simulink model "CCMHBMFMgr"  (R2022b)
settings: Solver=FixedStepDiscrete  StartTime=0.0  StopTime=10.0  FixedStep=0.1  RelTol=1e-3
undefined symbols (not defined in the file or in its folder): NetworkChlType, P_LinIfSchHandleType
settings Model: Creator=blue.ge  LastModifiedBy=blue.ge
settings DataIOCC: SaveFormat=StructureWithTime
settings OptimizationCC: DefaultParameterBehavior=Inlined  EnhancedBackFolding=on  PassReuseOutputArgsAs=Individual arguments  ZeroExternalMemoryAtStartup=off  ZeroInternalMemoryAtStartup=off  OptimizeBlockOrder=speed  DifferentSizesBufferReuse=on  OptimizationCustomize=off  EfficientTunableParamExpr=on
settings DebuggingCC: ParameterTunabilityLossMsg=error
settings ModelReferenceCC: ModelReferenceNumInstancesAllowed=Single
settings SFSimCC: MATLABDynamicMemAlloc=off
settings RTWCC: Description=AUTOSAR  SystemTargetFile=autosar.tlc  GenCodeOnly=on  TemplateMakefile=ert_default_tmf
settings CodeAppCC: ForceParamTrailComments=on  IgnoreCustomStorageClasses=off  OperatorAnnotations=on  SimulinkDataObjDesc=on  SFDataObjDesc=on  InsertBlockDesc=on
settings STFCustomTargetCC: TargetLangStandard=C99 (ISO)  MultiwordLength=256  ParMdlRefBuildCompliant=on  CompOptLevelCompliant=on  IncludeMdlTerminateFcn=off  CombineOutputUpdateFcns=on  SuppressErrorStatus=on  ERTCustomFileBanners=on  SupportNonFinite=off  SupportComplex=off  SupportContinuousTime=off  SupportNonInlinedSFcns=off  RemoveResetFunc=on  MATLABClassNameForMDSCustomization=Simulink.SoftwareTarget.ERTCustomization  AutosarCompliant=on  UseToolchainInfoCompliant=on  LookupTableObjectStructAxisOrder=2,1,3,4,...  LUTObjectStructOrderEvenSpacing=Size,Table,Breakpoints  SystemTargetFile=autosar.tlc
settings ERTTargetCC: TargetLangStandard=C99 (ISO)  ConcurrentExecutionCompliant=off  IncludeMdlTerminateFcn=off  SuppressErrorStatus=on  SupportNonFinite=off  SupportComplex=off  ModelStepFunctionPrototypeControlCompliant=off  CPPClassGenCompliant=off  AutosarCompliant=on  LookupTableObjectStructAxisOrder=2,1,3,4,...  LUTObjectStructOrderEvenSpacing=Size,Table,Breakpoints  InlinedParameterPlacement=NonHierarchical  ERTCustomFileTemplate=example_file_process.tlc

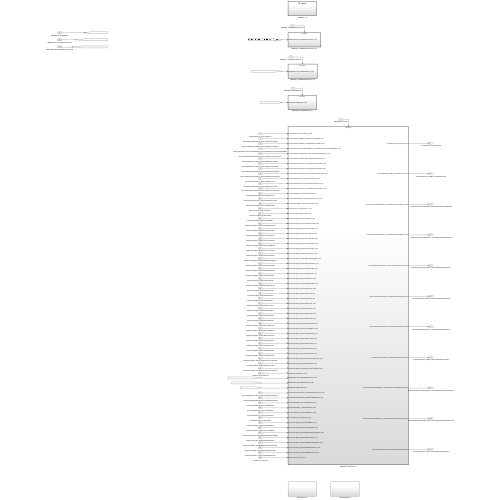
[thumbnail: Simulink's stored preview of the top-level diagram]
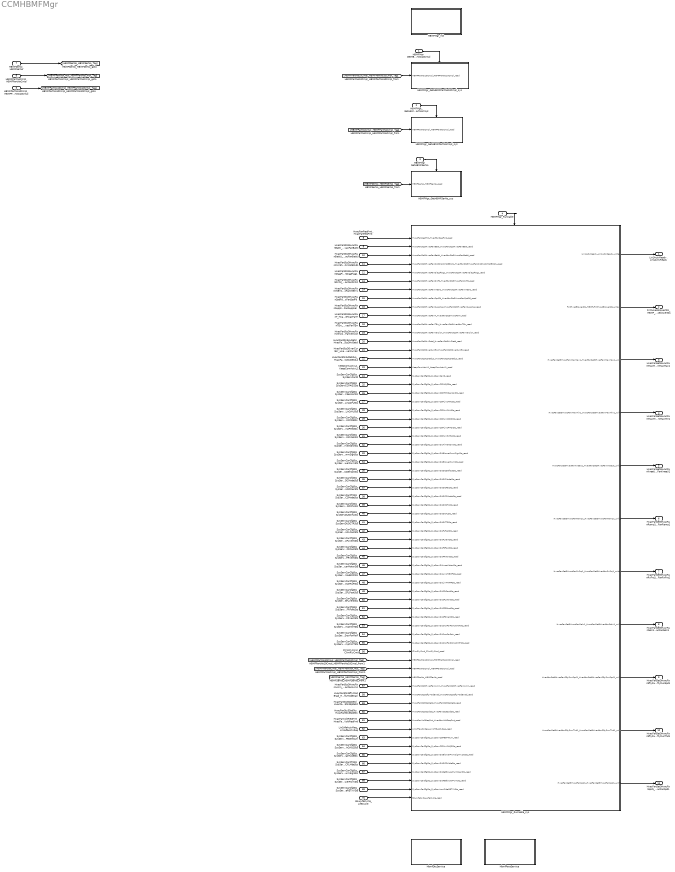
== system CCMHBMFMgr ==
  HBMFMgr_GetHBMFPartNo10Cmpl : Inport  OutputFunctionCall=on
  HBMFMgr_GetHBMFPartNoCmpl : Inport  Port=2  OutputFunctionCall=on
  HBMFMgr_GetHBMFSerNo : Inport  Port=3  OutputFunctionCall=on
  HBMFMgr_Runnable : Inport  Port=4  SampleTime=0.1  OutputFunctionCall=on
  HBMFPartNo10Cmpl_HBMFPartNo10Cmpl : Inport  Port=5  OutDataTypeStr=Bus: PartNrNod10  PortDimensions=1  BusOutputAsStruct=on
  HBMFPartNoCmpl_HBMFPartNoCmpl : Inport  Port=6  OutDataTypeStr=Bus: PartNrNodLIN  PortDimensions=1  BusOutputAsStruct=on
  HBMFSerNo_HBMFSerNo : Inport  Port=7  OutDataTypeStr=Bus: NrSerlNodLIN  PortDimensions=1  BusOutputAsStruct=on
  HvacFanReqFrnt_HvacFanReqFrnt : Inport  Port=8  OutDataTypeStr=HvacFanSpd  PortDimensions=1
  HvacFanSts3HvacFanBattI_HvacFanSts3HvacFanBattI : Inport  Port=9  OutDataTypeStr=BlowerBattI  PortDimensions=1
  HvacFanSts3HvacFanBattU_HvacFanSts3HvacFanBattU : Inport  Port=10  OutDataTypeStr=U10  PortDimensions=1
  HvacFanSts3HvacFanCircShoOrMotBlkd1_HvacFanSts3HvacFanCircShoOrMotBlkd1 : Inport  Port=11  OutDataTypeStr=HvacFanCircShoOrMotBlkdTyp  PortDimensions=1
  HvacFanSts3HvacFanDiagFlag1_HvacFanSts3HvacFanDiagFlag1 : Inport  Port=12  OutDataTypeStr=Enum: HvacFanDiagFlag  PortDimensions=1
  HvacFanSts3HvacFanDirFd_HvacFanSts3HvacFanDirFd : Inport  Port=13  OutDataTypeStr=Enum: HvacFanDir  PortDimensions=1
  HvacFanSts3HvacFanIntErr1_HvacFanSts3HvacFanIntErr1 : Inport  Port=14  OutDataTypeStr=HvacFanIntErrTyp  PortDimensions=1
  HvacFanSts3HvacFanSpdFd_HvacFanSts3HvacFanSpdFd : Inport  Port=15  OutDataTypeStr=BlowerSpd  PortDimensions=1
  HvacFanSts3HvacFanSupplier_HvacFanSts3HvacFanSupplier : Inport  Port=16  OutMin=0  OutMax=15  OutDataTypeStr=UInt4  PortDimensions=1
  HvacFanSts3HvacFanT_HvacFanSts3HvacFanT : Inport  Port=17  OutDataTypeStr=T6  PortDimensions=1
  HvacFanSts3HvacFanTErr_HvacFanSts3HvacFanTErr : Inport  Port=18  OutDataTypeStr=Boolean  PortDimensions=1
  HvacFanSts3HvacFanVersion_HvacFanSts3HvacFanVersion : Inport  Port=19  OutDataTypeStr=UInt8  PortDimensions=1
  HvacFanSts3HvDetd_HvacFanSts3HvDetd : Inport  Port=20  OutDataTypeStr=Boolean  PortDimensions=1
  HvacFanSts3OverCurrErr_HvacFanSts3OverCurrErr : Inport  Port=21  OutDataTypeStr=Boolean  PortDimensions=1
  HvacFanSts3UDetdLo_HvacFanSts3UDetdLo : Inport  Port=22  OutDataTypeStr=Boolean  PortDimensions=1
  KeepComActvL1_KeepComActvL1 : Inport  Port=23  OutDataTypeStr=Enum: OnOff1  PortDimensions=1
  SysServCarCfgSts_SysServCarID : Inport  Port=24  OutDataTypeStr=Enum: SysServCarID  PortDimensions=1
  SysServCarCfgSts_SysServCCPAQSSts : Inport  Port=25  OutDataTypeStr=UInt8  PortDimensions=1
  SysServCarCfgSts_SysServCCPHEXSensorSts : Inport  Port=26  OutDataTypeStr=UInt8  PortDimensions=1
  SysServCarCfgSts_SysServCCPLin2AFUSts : Inport  Port=27  OutDataTypeStr=Boolean  PortDimensions=1
  SysServCarCfgSts_SysServCCPLin2AIUSts : Inport  Port=28  OutDataTypeStr=UInt8  PortDimensions=1
  SysServCarCfgSts_SysServCCPLin2CDSSts : Inport  Port=29  OutDataTypeStr=Boolean  PortDimensions=1
  SysServCarCfgSts_SysServCCPLin2PMSISts : Inport  Port=30  OutDataTypeStr=Boolean  PortDimensions=1
  SysServCarCfgSts_SysServCCPLin3VGASts : Inport  Port=31  OutDataTypeStr=Boolean  PortDimensions=1
  SysServCarCfgSts_SysServDUAirVentSnrSts : Inport  Port=32  OutDataTypeStr=Boolean  PortDimensions=1
  SysServCarCfgSts_SysServDUBlowerPwmSignSts : Inport  Port=33  OutDataTypeStr=Boolean  PortDimensions=1
  SysServCarCfgSts_SysServDUBlowerPwmSts : Inport  Port=34  OutDataTypeStr=Boolean  PortDimensions=1
  SysServCarCfgSts_SysServDUDCDefrostSts : Inport  Port=35  OutDataTypeStr=UInt8  PortDimensions=1
  SysServCarCfgSts_SysServDUDCModeSts : Inport  Port=36  OutDataTypeStr=UInt8  PortDimensions=1
  SysServCarCfgSts_SysServDUDCRecSts : Inport  Port=37  OutDataTypeStr=UInt8  PortDimensions=1
  SysServCarCfgSts_SysServDUDCSModeSts : Inport  Port=38  OutDataTypeStr=UInt8  PortDimensions=1
  SysServCarCfgSts_SysServDUDCSTLSts : Inport  Port=39  OutDataTypeStr=UInt8  PortDimensions=1
  SysServCarCfgSts_SysServDUDCTLSts : Inport  Port=40  OutDataTypeStr=UInt8  PortDimensions=1
  SysServCarCfgSts_SysServDUDCTRSts : Inport  Port=41  OutDataTypeStr=UInt8  PortDimensions=1
  SysServCarCfgSts_SysServDUFLFootSts : Inport  Port=42  OutDataTypeStr=UInt8  PortDimensions=1
  SysServCarCfgSts_SysServDUFLVentSts : Inport  Port=43  OutDataTypeStr=UInt8  PortDimensions=1
  SysServCarCfgSts_SysServDUFRFootSts : Inport  Port=44  OutDataTypeStr=UInt8  PortDimensions=1
  SysServCarCfgSts_SysServDUFRVentSts : Inport  Port=45  OutDataTypeStr=UInt8  PortDimensions=1
  SysServCarCfgSts_SysServDUIncarMotorSts : Inport  Port=46  OutDataTypeStr=Boolean  PortDimensions=1
  SysServCarCfgSts_SysServDULin1HBMFSts : Inport  Port=47  OutDataTypeStr=Boolean  PortDimensions=1
  SysServCarCfgSts_SysServDULin4HFAFSts : Inport  Port=48  OutDataTypeStr=UInt32  PortDimensions=1
  SysServCarCfgSts_SysServDUSFLFootSts : Inport  Port=49  OutDataTypeStr=UInt8  PortDimensions=1
  SysServCarCfgSts_SysServDUSFLVentSts : Inport  Port=50  OutDataTypeStr=UInt8  PortDimensions=1
  SysServCarCfgSts_SysServDUSFRFootSts : Inport  Port=51  OutDataTypeStr=UInt8  PortDimensions=1
  SysServCarCfgSts_SysServDUSFRVentSts : Inport  Port=52  OutDataTypeStr=UInt8  PortDimensions=1
  SysServCarCfgSts_SysServDUSnsrForFCmptmtTSts : Inport  Port=53  OutDataTypeStr=UInt8  PortDimensions=1
  SysServCarCfgSts_SysServDUSnsrForRair : Inport  Port=54  OutDataTypeStr=UInt8  PortDimensions=1
  SysServCarCfgSts_SysServDUSnsrForRCmptmtTSts : Inport  Port=55  OutDataTypeStr=UInt8  PortDimensions=1
  ClimRlyCmd_ClimRlyCmd : Inport  Port=56  OutDataTypeStr=Enum: OnOff1  PortDimensions=1
  HvacFanSts3HvacFanILimn_HvacFanSts3HvacFanILimn : Inport  Port=57  OutDataTypeStr=Boolean  PortDimensions=1
  HvacFanSts3SftyModEnad_HvacFanSts3SftyModEnad : Inport  Port=58  OutDataTypeStr=Boolean  PortDimensions=1
  HvacFanSts3SpDetd_HvacFanSts3SpDetd : Inport  Port=59  OutDataTypeStr=Boolean  PortDimensions=1
  HvacFanSts3SpdSts_HvacFanSts3SpdSts : Inport  Port=60  OutDataTypeStr=Boolean  PortDimensions=1
  HvacFanVoltReqFrnt_HvacFanVoltReqFrnt : Inport  Port=61  OutMin=0  OutMax=100  OutDataTypeStr=Perc9  PortDimensions=1
  Lin1HfaActvReq_Lin1HfaActvReq : Inport  Port=62  OutDataTypeStr=UInt8  PortDimensions=1
  SysServCarCfgSts_SysServCCPBGMNum : Inport  Port=63  OutDataTypeStr=UInt8  PortDimensions=1
  SysServCarCfgSts_SysServCCPLin2AQSSts : Inport  Port=64  OutDataTypeStr=Boolean  PortDimensions=1
  SysServCarCfgSts_SysServDUBlowerPwmSignTypeSts : Inport  Port=65  OutDataTypeStr=UInt8  PortDimensions=1
  SysServCarCfgSts_SysServDUDCFLMdeSts : Inport  Port=66  OutDataTypeStr=UInt8  PortDimensions=1
  SysServCarCfgSts_SysServDUReBlowerPwmSignSts : Inport  Port=67  OutDataTypeStr=UInt8  PortDimensions=1
  SysServCarCfgSts_SysServDUReBlowerPwmSts : Inport  Port=68  OutDataTypeStr=UInt8  PortDimensions=1
  SysServCarCfgSts_SysServLowSideRETXVSts : Inport  Port=69  OutDataTypeStr=UInt8  PortDimensions=1
  BswLifeCycle_LifeCycle : Inport  Port=70  OutDataTypeStr=Enum: CCMLifeCycleModeGroup  PortDimensions=1
  HBMFMgr_GetHBMFPartNo10Cmpl_sys : Subsystem
  HBMFMgr_GetHBMFPartNoCmpl_sys : Subsystem
  HBMFMgr_GetHBMFSerNo_sys : Subsystem
  HBMFMgr_Init : Subsystem
  HBMFMgr_Runnable_sys : Subsystem
  HbmfDtcService : Subsystem
  HbmfParaService : Subsystem
  Lin1ActvReady_Lin1ActvReady : Outport  OutDataTypeStr=UInt8  PortDimensions=1
  FrntHvacBlowerSts_HBMF_FrntHvacBlowerSts : Outport  Port=2  OutDataTypeStr=Enum: HvacFanSts  PortDimensions=1
  HvacFanSet3HvacFanIMaxMax1_HvacFanSet3HvacFanIMaxMax1 : Outport  Port=3  OutDataTypeStr=BlowerIMAX1  PortDimensions=1
  HvacFanSet3HvacFanIMaxMin1_HvacFanSet3HvacFanIMaxMin1 : Outport  Port=4  OutDataTypeStr=Ampr3  PortDimensions=1
  HvacFanSet3HvacFanKneeU1_HvacFanSet3HvacFanKneeU1 : Outport  Port=5  OutDataTypeStr=U53  PortDimensions=1
  HvacFanSet3HvacFanRamp1_HvacFanSet3HvacFanRamp1 : Outport  Port=6  OutDataTypeStr=BlowerRamp  PortDimensions=1
  HvacFanSet3HvacFanRxFrq1_HvacFanSet3HvacFanRxFrq1 : Outport  Port=7  OutDataTypeStr=Uint12  PortDimensions=1
  HvacFanSet3HvacFanSeln1_HvacFanSet3HvacFanSeln1 : Outport  Port=8  OutDataTypeStr=Enum: HvacFanSeln  PortDimensions=1
  HvacFanSet3HvacFanSftyRunSpd1_HvacFanSet3HvacFanSftyRunSpd1 : Outport  Port=9  OutDataTypeStr=BlowerSpd  PortDimensions=1
  HvacFanSet3HvacFanSftyRunThd1_HvacFanSet3HvacFanSftyRunThd1 : Outport  Port=10  OutDataTypeStr=HvacFanSftyRunThd  PortDimensions=1
  HvacFanSet3HvacFanSpd1_HvacFanSet3HvacFanSpd1 : Outport  Port=11  OutDataTypeStr=BlowerSpd  PortDimensions=1
  wires:
  HvacFanReqFrnt_HvacFanReqFrnt/1 -> HBMFMgr_Runnable_sys/1(HvacFanReqFrnt_HvacFanReqFrnt_read)
  HvacFanSts3HvacFanBattI_HvacFanSts3HvacFanBattI/1 -> HBMFMgr_Runnable_sys/2(HvacFanSts3HvacFanBattI_HvacFanSts3HvacFanBattI_read)
  HvacFanSts3HvacFanBattU_HvacFanSts3HvacFanBattU/1 -> HBMFMgr_Runnable_sys/3(HvacFanSts3HvacFanBattU_HvacFanSts3HvacFanBattU_read)
  HvacFanSts3HvacFanCircShoOrMotBlkd1_HvacFanSts3HvacFanCircShoOrMotBlkd1/1 -> HBMFMgr_Runnable_sys/4(HvacFanSts3HvacFanCircShoOrMotBlkd1_HvacFanSts3HvacFanCircShoOrMotBlkd1_read)
  HvacFanSts3HvacFanDiagFlag1_HvacFanSts3HvacFanDiagFlag1/1 -> HBMFMgr_Runnable_sys/5(HvacFanSts3HvacFanDiagFlag1_HvacFanSts3HvacFanDiagFlag1_read)
  HvacFanSts3HvacFanDirFd_HvacFanSts3HvacFanDirFd/1 -> HBMFMgr_Runnable_sys/6(HvacFanSts3HvacFanDirFd_HvacFanSts3HvacFanDirFd_read)
  HvacFanSts3HvacFanIntErr1_HvacFanSts3HvacFanIntErr1/1 -> HBMFMgr_Runnable_sys/7(HvacFanSts3HvacFanIntErr1_HvacFanSts3HvacFanIntErr1_read)
  HvacFanSts3HvacFanSpdFd_HvacFanSts3HvacFanSpdFd/1 -> HBMFMgr_Runnable_sys/8(HvacFanSts3HvacFanSpdFd_HvacFanSts3HvacFanSpdFd_read)
  HvacFanSts3HvacFanSupplier_HvacFanSts3HvacFanSupplier/1 -> HBMFMgr_Runnable_sys/9(HvacFanSts3HvacFanSupplier_HvacFanSts3HvacFanSupplier_read)
  HvacFanSts3HvacFanT_HvacFanSts3HvacFanT/1 -> HBMFMgr_Runnable_sys/10(HvacFanSts3HvacFanT_HvacFanSts3HvacFanT_read)
  HvacFanSts3HvacFanTErr_HvacFanSts3HvacFanTErr/1 -> HBMFMgr_Runnable_sys/11(HvacFanSts3HvacFanTErr_HvacFanSts3HvacFanTErr_read)
  HvacFanSts3HvacFanVersion_HvacFanSts3HvacFanVersion/1 -> HBMFMgr_Runnable_sys/12(HvacFanSts3HvacFanVersion_HvacFanSts3HvacFanVersion_read)
  HvacFanSts3HvDetd_HvacFanSts3HvDetd/1 -> HBMFMgr_Runnable_sys/13(HvacFanSts3HvDetd_HvacFanSts3HvDetd_read)
  HvacFanSts3OverCurrErr_HvacFanSts3OverCurrErr/1 -> HBMFMgr_Runnable_sys/14(HvacFanSts3OverCurrErr_HvacFanSts3OverCurrErr_read)
  HvacFanSts3UDetdLo_HvacFanSts3UDetdLo/1 -> HBMFMgr_Runnable_sys/15(HvacFanSts3UDetdLo_HvacFanSts3UDetdLo_read)
  KeepComActvL1_KeepComActvL1/1 -> HBMFMgr_Runnable_sys/16(KeepComActvL1_KeepComActvL1_read)
  SysServCarCfgSts_SysServCarID/1 -> HBMFMgr_Runnable_sys/17(SysServCarCfgSts_SysServCarID_read)
  SysServCarCfgSts_SysServCCPAQSSts/1 -> HBMFMgr_Runnable_sys/18(SysServCarCfgSts_SysServCCPAQSSts_read)
  SysServCarCfgSts_SysServCCPHEXSensorSts/1 -> HBMFMgr_Runnable_sys/19(SysServCarCfgSts_SysServCCPHEXSensorSts_read)
  SysServCarCfgSts_SysServCCPLin2AFUSts/1 -> HBMFMgr_Runnable_sys/20(SysServCarCfgSts_SysServCCPLin2AFUSts_read)
  SysServCarCfgSts_SysServCCPLin2AIUSts/1 -> HBMFMgr_Runnable_sys/21(SysServCarCfgSts_SysServCCPLin2AIUSts_read)
  SysServCarCfgSts_SysServCCPLin2CDSSts/1 -> HBMFMgr_Runnable_sys/22(SysServCarCfgSts_SysServCCPLin2CDSSts_read)
  SysServCarCfgSts_SysServCCPLin2PMSISts/1 -> HBMFMgr_Runnable_sys/23(SysServCarCfgSts_SysServCCPLin2PMSISts_read)
  SysServCarCfgSts_SysServCCPLin3VGASts/1 -> HBMFMgr_Runnable_sys/24(SysServCarCfgSts_SysServCCPLin3VGASts_read)
  SysServCarCfgSts_SysServDUAirVentSnrSts/1 -> HBMFMgr_Runnable_sys/25(SysServCarCfgSts_SysServDUAirVentSnrSts_read)
  SysServCarCfgSts_SysServDUBlowerPwmSignSts/1 -> HBMFMgr_Runnable_sys/26(SysServCarCfgSts_SysServDUBlowerPwmSignSts_read)
  SysServCarCfgSts_SysServDUBlowerPwmSts/1 -> HBMFMgr_Runnable_sys/27(SysServCarCfgSts_SysServDUBlowerPwmSts_read)
  SysServCarCfgSts_SysServDUDCDefrostSts/1 -> HBMFMgr_Runnable_sys/28(SysServCarCfgSts_SysServDUDCDefrostSts_read)
  SysServCarCfgSts_SysServDUDCModeSts/1 -> HBMFMgr_Runnable_sys/29(SysServCarCfgSts_SysServDUDCModeSts_read)
  SysServCarCfgSts_SysServDUDCRecSts/1 -> HBMFMgr_Runnable_sys/30(SysServCarCfgSts_SysServDUDCRecSts_read)
  SysServCarCfgSts_SysServDUDCSModeSts/1 -> HBMFMgr_Runnable_sys/31(SysServCarCfgSts_SysServDUDCSModeSts_read)
  SysServCarCfgSts_SysServDUDCSTLSts/1 -> HBMFMgr_Runnable_sys/32(SysServCarCfgSts_SysServDUDCSTLSts_read)
  SysServCarCfgSts_SysServDUDCTLSts/1 -> HBMFMgr_Runnable_sys/33(SysServCarCfgSts_SysServDUDCTLSts_read)
  SysServCarCfgSts_SysServDUDCTRSts/1 -> HBMFMgr_Runnable_sys/34(SysServCarCfgSts_SysServDUDCTRSts_read)
  SysServCarCfgSts_SysServDUFLFootSts/1 -> HBMFMgr_Runnable_sys/35(SysServCarCfgSts_SysServDUFLFootSts_read)
  SysServCarCfgSts_SysServDUFLVentSts/1 -> HBMFMgr_Runnable_sys/36(SysServCarCfgSts_SysServDUFLVentSts_read)
  SysServCarCfgSts_SysServDUFRFootSts/1 -> HBMFMgr_Runnable_sys/37(SysServCarCfgSts_SysServDUFRFootSts_read)
  SysServCarCfgSts_SysServDUFRVentSts/1 -> HBMFMgr_Runnable_sys/38(SysServCarCfgSts_SysServDUFRVentSts_read)
  SysServCarCfgSts_SysServDUIncarMotorSts/1 -> HBMFMgr_Runnable_sys/39(SysServCarCfgSts_SysServDUIncarMotorSts_read)
  SysServCarCfgSts_SysServDULin1HBMFSts/1 -> HBMFMgr_Runnable_sys/40(SysServCarCfgSts_SysServDULin1HBMFSts_read)
  SysServCarCfgSts_SysServDULin4HFAFSts/1 -> HBMFMgr_Runnable_sys/41(SysServCarCfgSts_SysServDULin4HFAFSts_read)
  SysServCarCfgSts_SysServDUSFLFootSts/1 -> HBMFMgr_Runnable_sys/42(SysServCarCfgSts_SysServDUSFLFootSts_read)
  SysServCarCfgSts_SysServDUSFLVentSts/1 -> HBMFMgr_Runnable_sys/43(SysServCarCfgSts_SysServDUSFLVentSts_read)
  SysServCarCfgSts_SysServDUSFRFootSts/1 -> HBMFMgr_Runnable_sys/44(SysServCarCfgSts_SysServDUSFRFootSts_read)
  SysServCarCfgSts_SysServDUSFRVentSts/1 -> HBMFMgr_Runnable_sys/45(SysServCarCfgSts_SysServDUSFRVentSts_read)
  SysServCarCfgSts_SysServDUSnsrForFCmptmtTSts/1 -> HBMFMgr_Runnable_sys/46(SysServCarCfgSts_SysServDUSnsrForFCmptmtTSts_read)
  SysServCarCfgSts_SysServDUSnsrForRair/1 -> HBMFMgr_Runnable_sys/47(SysServCarCfgSts_SysServDUSnsrForRair_read)
  SysServCarCfgSts_SysServDUSnsrForRCmptmtTSts/1 -> HBMFMgr_Runnable_sys/48(SysServCarCfgSts_SysServDUSnsrForRCmptmtTSts_read)
  ClimRlyCmd_ClimRlyCmd/1 -> HBMFMgr_Runnable_sys/49(ClimRlyCmd_ClimRlyCmd_read)
  HvacFanSts3HvacFanILimn_HvacFanSts3HvacFanILimn/1 -> HBMFMgr_Runnable_sys/53(HvacFanSts3HvacFanILimn_HvacFanSts3HvacFanILimn_read)
  HvacFanSts3SftyModEnad_HvacFanSts3SftyModEnad/1 -> HBMFMgr_Runnable_sys/54(HvacFanSts3SftyModEnad_HvacFanSts3SftyModEnad_read)
  HvacFanSts3SpDetd_HvacFanSts3SpDetd/1 -> HBMFMgr_Runnable_sys/55(HvacFanSts3SpDetd_HvacFanSts3SpDetd_read)
  HvacFanSts3SpdSts_HvacFanSts3SpdSts/1 -> HBMFMgr_Runnable_sys/56(HvacFanSts3SpdSts_HvacFanSts3SpdSts_read)
  HvacFanVoltReqFrnt_HvacFanVoltReqFrnt/1 -> HBMFMgr_Runnable_sys/57(HvacFanVoltReqFrnt_HvacFanVoltReqFrnt_read)
  Lin1HfaActvReq_Lin1HfaActvReq/1 -> HBMFMgr_Runnable_sys/58(Lin1HfaActvReq_Lin1HfaActvReq_read)
  SysServCarCfgSts_SysServCCPBGMNum/1 -> HBMFMgr_Runnable_sys/59(SysServCarCfgSts_SysServCCPBGMNum_read)
  SysServCarCfgSts_SysServCCPLin2AQSSts/1 -> HBMFMgr_Runnable_sys/60(SysServCarCfgSts_SysServCCPLin2AQSSts_read)
  SysServCarCfgSts_SysServDUBlowerPwmSignTypeSts/1 -> HBMFMgr_Runnable_sys/61(SysServCarCfgSts_SysServDUBlowerPwmSignTypeSts_read)
  SysServCarCfgSts_SysServDUDCFLMdeSts/1 -> HBMFMgr_Runnable_sys/62(SysServCarCfgSts_SysServDUDCFLMdeSts_read)
  SysServCarCfgSts_SysServDUReBlowerPwmSignSts/1 -> HBMFMgr_Runnable_sys/63(SysServCarCfgSts_SysServDUReBlowerPwmSignSts_read)
  SysServCarCfgSts_SysServDUReBlowerPwmSts/1 -> HBMFMgr_Runnable_sys/64(SysServCarCfgSts_SysServDUReBlowerPwmSts_read)
  SysServCarCfgSts_SysServLowSideRETXVSts/1 -> HBMFMgr_Runnable_sys/65(SysServCarCfgSts_SysServLowSideRETXVSts_read)
  HBMFMgr_Runnable_sys/1(Lin1ActvReady_Lin1ActvReady_write) -> Lin1ActvReady_Lin1ActvReady/1
  HBMFMgr_Runnable_sys/2(FrntHvacBlowerSts_HBMF_FrntHvacBlowerSts_write) -> FrntHvacBlowerSts_HBMF_FrntHvacBlowerSts/1
  HBMFMgr_Runnable_sys/3(HvacFanSet3HvacFanIMaxMax1_HvacFanSet3HvacFanIMaxMax1_write) -> HvacFanSet3HvacFanIMaxMax1_HvacFanSet3HvacFanIMaxMax1/1
  HBMFMgr_Runnable_sys/4(HvacFanSet3HvacFanIMaxMin1_HvacFanSet3HvacFanIMaxMin1_write) -> HvacFanSet3HvacFanIMaxMin1_HvacFanSet3HvacFanIMaxMin1/1
  HBMFMgr_Runnable_sys/5(HvacFanSet3HvacFanKneeU1_HvacFanSet3HvacFanKneeU1_write) -> HvacFanSet3HvacFanKneeU1_HvacFanSet3HvacFanKneeU1/1
  HBMFMgr_Runnable_sys/6(HvacFanSet3HvacFanRamp1_HvacFanSet3HvacFanRamp1_write) -> HvacFanSet3HvacFanRamp1_HvacFanSet3HvacFanRamp1/1
  HBMFMgr_Runnable_sys/7(HvacFanSet3HvacFanRxFrq1_HvacFanSet3HvacFanRxFrq1_write) -> HvacFanSet3HvacFanRxFrq1_HvacFanSet3HvacFanRxFrq1/1
  HBMFMgr_Runnable_sys/8(HvacFanSet3HvacFanSeln1_HvacFanSet3HvacFanSeln1_write) -> HvacFanSet3HvacFanSeln1_HvacFanSet3HvacFanSeln1/1
  HBMFMgr_Runnable_sys/9(HvacFanSet3HvacFanSftyRunSpd1_HvacFanSet3HvacFanSftyRunSpd1_write) -> HvacFanSet3HvacFanSftyRunSpd1_HvacFanSet3HvacFanSftyRunSpd1/1
  HBMFMgr_Runnable_sys/10(HvacFanSet3HvacFanSftyRunThd1_HvacFanSet3HvacFanSftyRunThd1_write) -> HvacFanSet3HvacFanSftyRunThd1_HvacFanSet3HvacFanSftyRunThd1/1
  HBMFMgr_Runnable_sys/11(HvacFanSet3HvacFanSpd1_HvacFanSet3HvacFanSpd1_write) -> HvacFanSet3HvacFanSpd1_HvacFanSet3HvacFanSpd1/1
  BswLifeCycle_LifeCycle/1 -> HBMFMgr_Runnable_sys/66(BswLifeCycle_LifeCycle_read)
  HBMFSerNo_HBMFSerNo/1 -> [HBMFSerNo_HBMFSerNo_Tag]
  [HBMFSerNo_HBMFSerNo_Tag] -> HBMFMgr_GetHBMFSerNo_sys/1(HBMFSerNo_HBMFSerNo_read)
  [HBMFSerNo_HBMFSerNo_Tag] -> HBMFMgr_Runnable_sys/52(HBMFSerNo_HBMFSerNo_read)
  HBMFPartNoCmpl_HBMFPartNoCmpl/1 -> [HBMFPartNoCmpl_HBMFPartNoCmpl_Tag]
  [HBMFPartNoCmpl_HBMFPartNoCmpl_Tag] -> HBMFMgr_GetHBMFPartNoCmpl_sys/1(HBMFPartNoCmpl_HBMFPartNoCmpl_read)
  [HBMFPartNoCmpl_HBMFPartNoCmpl_Tag] -> HBMFMgr_Runnable_sys/51(HBMFPartNoCmpl_HBMFPartNoCmpl_read)
  HBMFPartNo10Cmpl_HBMFPartNo10Cmpl/1 -> [HBMFPartNo10Cmpl_HBMFPartNo10Cmpl_Tag]
  [HBMFPartNo10Cmpl_HBMFPartNo10Cmpl_Tag] -> HBMFMgr_GetHBMFPartNo10Cmpl_sys/1(HBMFPartNo10Cmpl_HBMFPartNo10Cmpl_read)
  [HBMFPartNo10Cmpl_HBMFPartNo10Cmpl_Tag] -> HBMFMgr_Runnable_sys/50(HBMFPartNo10Cmpl_HBMFPartNo10Cmpl_read)
  HBMFMgr_GetHBMFPartNo10Cmpl/1 -> HBMFMgr_GetHBMFPartNo10Cmpl_sys/Trigger
  HBMFMgr_GetHBMFPartNoCmpl/1 -> HBMFMgr_GetHBMFPartNoCmpl_sys/Trigger
  HBMFMgr_GetHBMFSerNo/1 -> HBMFMgr_GetHBMFSerNo_sys/Trigger
  HBMFMgr_Runnable/1 -> HBMFMgr_Runnable_sys/Trigger

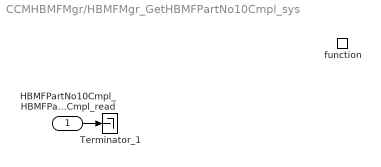
== system CCMHBMFMgr/HBMFMgr_GetHBMFPartNo10Cmpl_sys ==
  HBMFPartNo10Cmpl_HBMFPartNo10Cmpl_read : Inport
  function : TriggerPort  TriggerType=function-call  VariantControl=(inherit)
  Terminator_1 : Terminator
  wires:
  HBMFPartNo10Cmpl_HBMFPartNo10Cmpl_read/1 -> Terminator_1/1

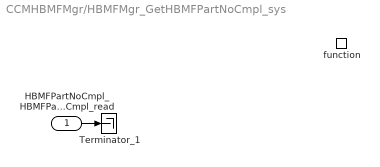
== system CCMHBMFMgr/HBMFMgr_GetHBMFPartNoCmpl_sys ==
  HBMFPartNoCmpl_HBMFPartNoCmpl_read : Inport
  function : TriggerPort  TriggerType=function-call  VariantControl=(inherit)
  Terminator_1 : Terminator
  wires:
  HBMFPartNoCmpl_HBMFPartNoCmpl_read/1 -> Terminator_1/1

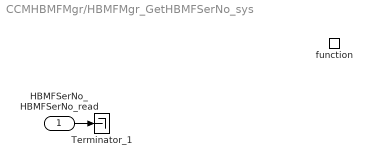
== system CCMHBMFMgr/HBMFMgr_GetHBMFSerNo_sys ==
  HBMFSerNo_HBMFSerNo_read : Inport
  function : TriggerPort  TriggerType=function-call  VariantControl=(inherit)
  Terminator_1 : Terminator
  wires:
  HBMFSerNo_HBMFSerNo_read/1 -> Terminator_1/1

== system CCMHBMFMgr/HBMFMgr_Init ==
  Event Listener : EventListener  EventName=reset

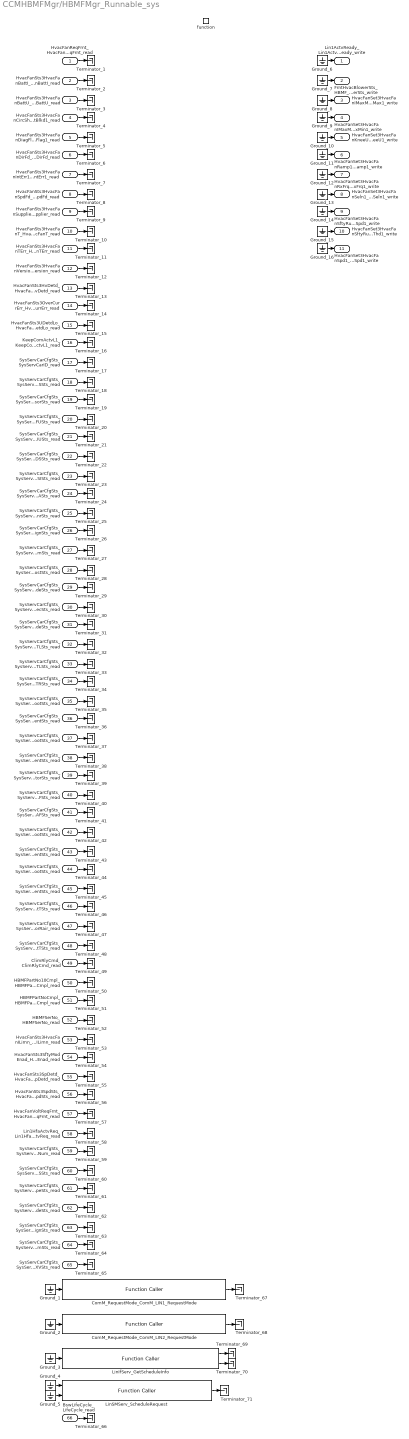
== system CCMHBMFMgr/HBMFMgr_Runnable_sys ==
  HvacFanReqFrnt_HvacFanReqFrnt_read : Inport
  HvacFanSts3HvacFanBattI_HvacFanSts3HvacFanBattI_read : Inport  Port=2
  HvacFanSts3HvacFanBattU_HvacFanSts3HvacFanBattU_read : Inport  Port=3
  HvacFanSts3HvacFanCircShoOrMotBlkd1_HvacFanSts3HvacFanCircShoOrMotBlkd1_read : Inport  Port=4
  HvacFanSts3HvacFanDiagFlag1_HvacFanSts3HvacFanDiagFlag1_read : Inport  Port=5
  HvacFanSts3HvacFanDirFd_HvacFanSts3HvacFanDirFd_read : Inport  Port=6
  HvacFanSts3HvacFanIntErr1_HvacFanSts3HvacFanIntErr1_read : Inport  Port=7
  HvacFanSts3HvacFanSpdFd_HvacFanSts3HvacFanSpdFd_read : Inport  Port=8
  HvacFanSts3HvacFanSupplier_HvacFanSts3HvacFanSupplier_read : Inport  Port=9
  HvacFanSts3HvacFanT_HvacFanSts3HvacFanT_read : Inport  Port=10
  HvacFanSts3HvacFanTErr_HvacFanSts3HvacFanTErr_read : Inport  Port=11
  HvacFanSts3HvacFanVersion_HvacFanSts3HvacFanVersion_read : Inport  Port=12
  HvacFanSts3HvDetd_HvacFanSts3HvDetd_read : Inport  Port=13
  HvacFanSts3OverCurrErr_HvacFanSts3OverCurrErr_read : Inport  Port=14
  HvacFanSts3UDetdLo_HvacFanSts3UDetdLo_read : Inport  Port=15
  KeepComActvL1_KeepComActvL1_read : Inport  Port=16
  SysServCarCfgSts_SysServCarID_read : Inport  Port=17
  SysServCarCfgSts_SysServCCPAQSSts_read : Inport  Port=18
  SysServCarCfgSts_SysServCCPHEXSensorSts_read : Inport  Port=19
  SysServCarCfgSts_SysServCCPLin2AFUSts_read : Inport  Port=20
  SysServCarCfgSts_SysServCCPLin2AIUSts_read : Inport  Port=21
  SysServCarCfgSts_SysServCCPLin2CDSSts_read : Inport  Port=22
  SysServCarCfgSts_SysServCCPLin2PMSISts_read : Inport  Port=23
  SysServCarCfgSts_SysServCCPLin3VGASts_read : Inport  Port=24
  SysServCarCfgSts_SysServDUAirVentSnrSts_read : Inport  Port=25
  SysServCarCfgSts_SysServDUBlowerPwmSignSts_read : Inport  Port=26
  SysServCarCfgSts_SysServDUBlowerPwmSts_read : Inport  Port=27
  SysServCarCfgSts_SysServDUDCDefrostSts_read : Inport  Port=28
  SysServCarCfgSts_SysServDUDCModeSts_read : Inport  Port=29
  SysServCarCfgSts_SysServDUDCRecSts_read : Inport  Port=30
  SysServCarCfgSts_SysServDUDCSModeSts_read : Inport  Port=31
  SysServCarCfgSts_SysServDUDCSTLSts_read : Inport  Port=32
  SysServCarCfgSts_SysServDUDCTLSts_read : Inport  Port=33
  SysServCarCfgSts_SysServDUDCTRSts_read : Inport  Port=34
  SysServCarCfgSts_SysServDUFLFootSts_read : Inport  Port=35
  SysServCarCfgSts_SysServDUFLVentSts_read : Inport  Port=36
  SysServCarCfgSts_SysServDUFRFootSts_read : Inport  Port=37
  SysServCarCfgSts_SysServDUFRVentSts_read : Inport  Port=38
  SysServCarCfgSts_SysServDUIncarMotorSts_read : Inport  Port=39
  SysServCarCfgSts_SysServDULin1HBMFSts_read : Inport  Port=40
  SysServCarCfgSts_SysServDULin4HFAFSts_read : Inport  Port=41
  SysServCarCfgSts_SysServDUSFLFootSts_read : Inport  Port=42
  SysServCarCfgSts_SysServDUSFLVentSts_read : Inport  Port=43
  SysServCarCfgSts_SysServDUSFRFootSts_read : Inport  Port=44
  SysServCarCfgSts_SysServDUSFRVentSts_read : Inport  Port=45
  SysServCarCfgSts_SysServDUSnsrForFCmptmtTSts_read : Inport  Port=46
  SysServCarCfgSts_SysServDUSnsrForRair_read : Inport  Port=47
  SysServCarCfgSts_SysServDUSnsrForRCmptmtTSts_read : Inport  Port=48
  ClimRlyCmd_ClimRlyCmd_read : Inport  Port=49
  HBMFPartNo10Cmpl_HBMFPartNo10Cmpl_read : Inport  Port=50
  HBMFPartNoCmpl_HBMFPartNoCmpl_read : Inport  Port=51
  HBMFSerNo_HBMFSerNo_read : Inport  Port=52
  HvacFanSts3HvacFanILimn_HvacFanSts3HvacFanILimn_read : Inport  Port=53
  HvacFanSts3SftyModEnad_HvacFanSts3SftyModEnad_read : Inport  Port=54
  HvacFanSts3SpDetd_HvacFanSts3SpDetd_read : Inport  Port=55
  HvacFanSts3SpdSts_HvacFanSts3SpdSts_read : Inport  Port=56
  HvacFanVoltReqFrnt_HvacFanVoltReqFrnt_read : Inport  Port=57
  Lin1HfaActvReq_Lin1HfaActvReq_read : Inport  Port=58
  SysServCarCfgSts_SysServCCPBGMNum_read : Inport  Port=59
  SysServCarCfgSts_SysServCCPLin2AQSSts_read : Inport  Port=60
  SysServCarCfgSts_SysServDUBlowerPwmSignTypeSts_read : Inport  Port=61
  SysServCarCfgSts_SysServDUDCFLMdeSts_read : Inport  Port=62
  SysServCarCfgSts_SysServDUReBlowerPwmSignSts_read : Inport  Port=63
  SysServCarCfgSts_SysServDUReBlowerPwmSts_read : Inport  Port=64
  SysServCarCfgSts_SysServLowSideRETXVSts_read : Inport  Port=65
  BswLifeCycle_LifeCycle_read : Inport  Port=66
  function : TriggerPort  TriggerType=function-call  VariantControl=(inherit)  SampleTimeType=periodic  SampleTime=-1
  ComM_RequestMode_ComM_LIN1_RequestMode : FunctionCaller  FunctionPrototype=ERR = ComM_RequestMode_ComM_LIN1_RequestMode(ReqMode)  InputArgumentSpecifications=uint16(1)  OutputArgumentSpecifications=P_Std_ReturnType
  ComM_RequestMode_ComM_LIN2_RequestMode : FunctionCaller  FunctionPrototype=ERR = ComM_RequestMode_ComM_LIN2_RequestMode(ReqMode)  InputArgumentSpecifications=uint16(1)  OutputArgumentSpecifications=P_Std_ReturnType
  Ground_{1..16} (16 alike) : Ground
  LinIfServ_GetScheduleInfo : FunctionCaller  FunctionPrototype=[ScheduleInfo,ERR] = LinIfServ_GetScheduleInfo(Channel)  InputArgumentSpecifications=NetworkChlType(0)  OutputArgumentSpecifications=P_LinIfScheduleInfoType, P_Std_ReturnType
  LinSMServ_ScheduleRequest : FunctionCaller  FunctionPrototype=ERR = LinSMServ_ScheduleRequest(network,schedule)  InputArgumentSpecifications=NetworkChlType(0), P_LinIfSchHandleType  OutputArgumentSpecifications=P_Std_ReturnType
  Terminator_{1..71} (71 alike) : Terminator
  Lin1ActvReady_Lin1ActvReady_write : Outport
      settings: EnsureOutportIsVirtual=on
  FrntHvacBlowerSts_HBMF_FrntHvacBlowerSts_write : Outport  Port=2
      settings: EnsureOutportIsVirtual=on
  HvacFanSet3HvacFanIMaxMax1_HvacFanSet3HvacFanIMaxMax1_write : Outport  Port=3
      settings: EnsureOutportIsVirtual=on
  HvacFanSet3HvacFanIMaxMin1_HvacFanSet3HvacFanIMaxMin1_write : Outport  Port=4
      settings: EnsureOutportIsVirtual=on
  HvacFanSet3HvacFanKneeU1_HvacFanSet3HvacFanKneeU1_write : Outport  Port=5
      settings: EnsureOutportIsVirtual=on
  HvacFanSet3HvacFanRamp1_HvacFanSet3HvacFanRamp1_write : Outport  Port=6
      settings: EnsureOutportIsVirtual=on
  HvacFanSet3HvacFanRxFrq1_HvacFanSet3HvacFanRxFrq1_write : Outport  Port=7
      settings: EnsureOutportIsVirtual=on
  HvacFanSet3HvacFanSeln1_HvacFanSet3HvacFanSeln1_write : Outport  Port=8
      settings: EnsureOutportIsVirtual=on
  HvacFanSet3HvacFanSftyRunSpd1_HvacFanSet3HvacFanSftyRunSpd1_write : Outport  Port=9
      settings: EnsureOutportIsVirtual=on
  HvacFanSet3HvacFanSftyRunThd1_HvacFanSet3HvacFanSftyRunThd1_write : Outport  Port=10
      settings: EnsureOutportIsVirtual=on
  HvacFanSet3HvacFanSpd1_HvacFanSet3HvacFanSpd1_write : Outport  Port=11
      settings: EnsureOutportIsVirtual=on
  wires:
  HvacFanReqFrnt_HvacFanReqFrnt_read/1 -> Terminator_1/1
  HvacFanSts3HvacFanBattI_HvacFanSts3HvacFanBattI_read/1 -> Terminator_2/1
  HvacFanSts3HvacFanBattU_HvacFanSts3HvacFanBattU_read/1 -> Terminator_3/1
  HvacFanSts3HvacFanCircShoOrMotBlkd1_HvacFanSts3HvacFanCircShoOrMotBlkd1_read/1 -> Terminator_4/1
  HvacFanSts3HvacFanDiagFlag1_HvacFanSts3HvacFanDiagFlag1_read/1 -> Terminator_5/1
  HvacFanSts3HvacFanDirFd_HvacFanSts3HvacFanDirFd_read/1 -> Terminator_6/1
  HvacFanSts3HvacFanIntErr1_HvacFanSts3HvacFanIntErr1_read/1 -> Terminator_7/1
  HvacFanSts3HvacFanSpdFd_HvacFanSts3HvacFanSpdFd_read/1 -> Terminator_8/1
  HvacFanSts3HvacFanSupplier_HvacFanSts3HvacFanSupplier_read/1 -> Terminator_9/1
  HvacFanSts3HvacFanT_HvacFanSts3HvacFanT_read/1 -> Terminator_10/1
  HvacFanSts3HvacFanTErr_HvacFanSts3HvacFanTErr_read/1 -> Terminator_11/1
  HvacFanSts3HvacFanVersion_HvacFanSts3HvacFanVersion_read/1 -> Terminator_12/1
  HvacFanSts3HvDetd_HvacFanSts3HvDetd_read/1 -> Terminator_13/1
  HvacFanSts3OverCurrErr_HvacFanSts3OverCurrErr_read/1 -> Terminator_14/1
  HvacFanSts3UDetdLo_HvacFanSts3UDetdLo_read/1 -> Terminator_15/1
  KeepComActvL1_KeepComActvL1_read/1 -> Terminator_16/1
  SysServCarCfgSts_SysServCarID_read/1 -> Terminator_17/1
  SysServCarCfgSts_SysServCCPAQSSts_read/1 -> Terminator_18/1
  SysServCarCfgSts_SysServCCPHEXSensorSts_read/1 -> Terminator_19/1
  SysServCarCfgSts_SysServCCPLin2AFUSts_read/1 -> Terminator_20/1
  SysServCarCfgSts_SysServCCPLin2AIUSts_read/1 -> Terminator_21/1
  SysServCarCfgSts_SysServCCPLin2CDSSts_read/1 -> Terminator_22/1
  SysServCarCfgSts_SysServCCPLin2PMSISts_read/1 -> Terminator_23/1
  SysServCarCfgSts_SysServCCPLin3VGASts_read/1 -> Terminator_24/1
  SysServCarCfgSts_SysServDUAirVentSnrSts_read/1 -> Terminator_25/1
  SysServCarCfgSts_SysServDUBlowerPwmSignSts_read/1 -> Terminator_26/1
  SysServCarCfgSts_SysServDUBlowerPwmSts_read/1 -> Terminator_27/1
  SysServCarCfgSts_SysServDUDCDefrostSts_read/1 -> Terminator_28/1
  SysServCarCfgSts_SysServDUDCModeSts_read/1 -> Terminator_29/1
  SysServCarCfgSts_SysServDUDCRecSts_read/1 -> Terminator_30/1
  SysServCarCfgSts_SysServDUDCSModeSts_read/1 -> Terminator_31/1
  SysServCarCfgSts_SysServDUDCSTLSts_read/1 -> Terminator_32/1
  SysServCarCfgSts_SysServDUDCTLSts_read/1 -> Terminator_33/1
  SysServCarCfgSts_SysServDUDCTRSts_read/1 -> Terminator_34/1
  SysServCarCfgSts_SysServDUFLFootSts_read/1 -> Terminator_35/1
  SysServCarCfgSts_SysServDUFLVentSts_read/1 -> Terminator_36/1
  SysServCarCfgSts_SysServDUFRFootSts_read/1 -> Terminator_37/1
  SysServCarCfgSts_SysServDUFRVentSts_read/1 -> Terminator_38/1
  SysServCarCfgSts_SysServDUIncarMotorSts_read/1 -> Terminator_39/1
  SysServCarCfgSts_SysServDULin1HBMFSts_read/1 -> Terminator_40/1
  SysServCarCfgSts_SysServDULin4HFAFSts_read/1 -> Terminator_41/1
  SysServCarCfgSts_SysServDUSFLFootSts_read/1 -> Terminator_42/1
  SysServCarCfgSts_SysServDUSFLVentSts_read/1 -> Terminator_43/1
  SysServCarCfgSts_SysServDUSFRFootSts_read/1 -> Terminator_44/1
  SysServCarCfgSts_SysServDUSFRVentSts_read/1 -> Terminator_45/1
  SysServCarCfgSts_SysServDUSnsrForFCmptmtTSts_read/1 -> Terminator_46/1
  SysServCarCfgSts_SysServDUSnsrForRair_read/1 -> Terminator_47/1
  SysServCarCfgSts_SysServDUSnsrForRCmptmtTSts_read/1 -> Terminator_48/1
  ClimRlyCmd_ClimRlyCmd_read/1 -> Terminator_49/1
  HBMFPartNo10Cmpl_HBMFPartNo10Cmpl_read/1 -> Terminator_50/1
  HBMFPartNoCmpl_HBMFPartNoCmpl_read/1 -> Terminator_51/1
  HBMFSerNo_HBMFSerNo_read/1 -> Terminator_52/1
  HvacFanSts3HvacFanILimn_HvacFanSts3HvacFanILimn_read/1 -> Terminator_53/1
  HvacFanSts3SftyModEnad_HvacFanSts3SftyModEnad_read/1 -> Terminator_54/1
  HvacFanSts3SpDetd_HvacFanSts3SpDetd_read/1 -> Terminator_55/1
  HvacFanSts3SpdSts_HvacFanSts3SpdSts_read/1 -> Terminator_56/1
  HvacFanVoltReqFrnt_HvacFanVoltReqFrnt_read/1 -> Terminator_57/1
  Lin1HfaActvReq_Lin1HfaActvReq_read/1 -> Terminator_58/1
  SysServCarCfgSts_SysServCCPBGMNum_read/1 -> Terminator_59/1
  SysServCarCfgSts_SysServCCPLin2AQSSts_read/1 -> Terminator_60/1
  SysServCarCfgSts_SysServDUBlowerPwmSignTypeSts_read/1 -> Terminator_61/1
  SysServCarCfgSts_SysServDUDCFLMdeSts_read/1 -> Terminator_62/1
  SysServCarCfgSts_SysServDUReBlowerPwmSignSts_read/1 -> Terminator_63/1
  SysServCarCfgSts_SysServDUReBlowerPwmSts_read/1 -> Terminator_64/1
  SysServCarCfgSts_SysServLowSideRETXVSts_read/1 -> Terminator_65/1
  BswLifeCycle_LifeCycle_read/1 -> Terminator_66/1
  Ground_1/1 -> ComM_RequestMode_ComM_LIN1_RequestMode/1
  ComM_RequestMode_ComM_LIN1_RequestMode/1 -> Terminator_67/1
  Ground_2/1 -> ComM_RequestMode_ComM_LIN2_RequestMode/1
  ComM_RequestMode_ComM_LIN2_RequestMode/1 -> Terminator_68/1
  Ground_3/1 -> LinIfServ_GetScheduleInfo/1
  LinIfServ_GetScheduleInfo/1 -> Terminator_69/1
  LinIfServ_GetScheduleInfo/2 -> Terminator_70/1
  Ground_4/1 -> LinSMServ_ScheduleRequest/1
  Ground_5/1 -> LinSMServ_ScheduleRequest/2
  LinSMServ_ScheduleRequest/1 -> Terminator_71/1
  Ground_6/1 -> Lin1ActvReady_Lin1ActvReady_write/1
  Ground_7/1 -> FrntHvacBlowerSts_HBMF_FrntHvacBlowerSts_write/1
  Ground_8/1 -> HvacFanSet3HvacFanIMaxMax1_HvacFanSet3HvacFanIMaxMax1_write/1
  Ground_9/1 -> HvacFanSet3HvacFanIMaxMin1_HvacFanSet3HvacFanIMaxMin1_write/1
  Ground_10/1 -> HvacFanSet3HvacFanKneeU1_HvacFanSet3HvacFanKneeU1_write/1
  Ground_11/1 -> HvacFanSet3HvacFanRamp1_HvacFanSet3HvacFanRamp1_write/1
  Ground_12/1 -> HvacFanSet3HvacFanRxFrq1_HvacFanSet3HvacFanRxFrq1_write/1
  Ground_13/1 -> HvacFanSet3HvacFanSeln1_HvacFanSet3HvacFanSeln1_write/1
  Ground_14/1 -> HvacFanSet3HvacFanSftyRunSpd1_HvacFanSet3HvacFanSftyRunSpd1_write/1
  Ground_15/1 -> HvacFanSet3HvacFanSftyRunThd1_HvacFanSet3HvacFanSftyRunThd1_write/1
  Ground_16/1 -> HvacFanSet3HvacFanSpd1_HvacFanSet3HvacFanSpd1_write/1

== system CCMHBMFMgr/HbmfDtcService ==
  HbmfDtcService_GetLin1HvacFanCircShoOrMotBlkd1Err_sys : Subsystem
  HbmfDtcService_GetLin1HvacFanIntErr1_sys : Subsystem

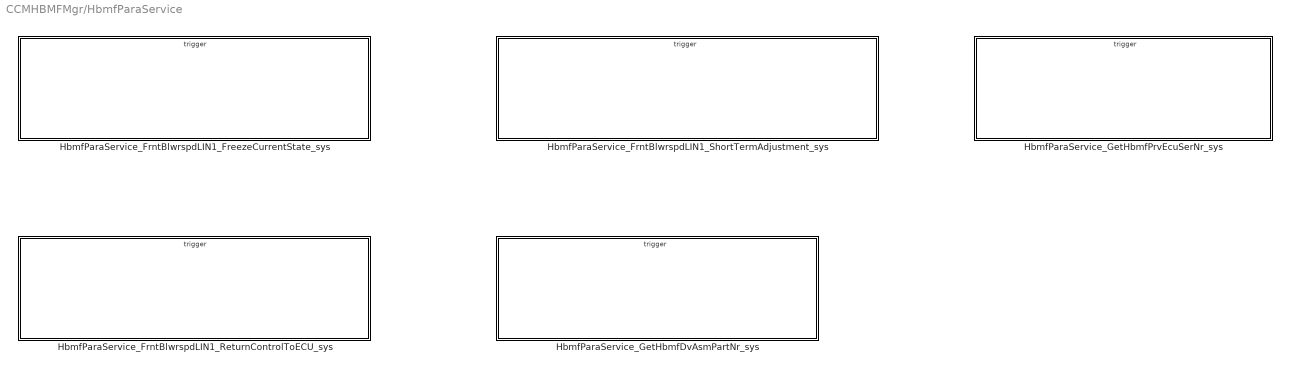
== system CCMHBMFMgr/HbmfParaService ==
  HbmfParaService_FrntBlwrspdLIN1_FreezeCurrentState_sys : Subsystem
  HbmfParaService_FrntBlwrspdLIN1_ReturnControlToECU_sys : Subsystem
  HbmfParaService_FrntBlwrspdLIN1_ShortTermAdjustment_sys : Subsystem
  HbmfParaService_GetHbmfDvAsmPartNr_sys : Subsystem
  HbmfParaService_GetHbmfPrvEcuSerNr_sys : Subsystem

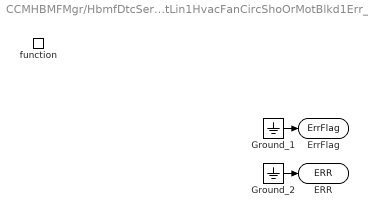
== system CCMHBMFMgr/HbmfDtcService/HbmfDtcService_GetLin1HvacFanCircShoOrMotBlkd1Err_sys ==
  function : TriggerPort  TriggerType=function-call  IsSimulinkFunction=on  FunctionName=HbmfDtcService_GetLin1HvacFanCircShoOrMotBlkd1Err  VariantControl=(inherit)
  Ground_1 : Ground
  Ground_2 : Ground
  ErrFlag : ArgOut  OutDataTypeStr=Enum: DTCState  ArgumentName=ErrFlag
  ERR : ArgOut  Port=2  OutDataTypeStr=Std_ReturnType  ArgumentName=ERR
  wires:
  Ground_1/1 -> ErrFlag/1
  Ground_2/1 -> ERR/1

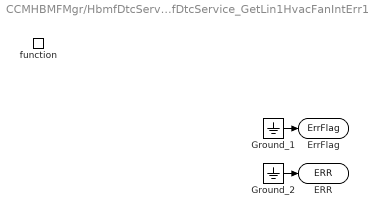
== system CCMHBMFMgr/HbmfDtcService/HbmfDtcService_GetLin1HvacFanIntErr1_sys ==
  function : TriggerPort  TriggerType=function-call  IsSimulinkFunction=on  FunctionName=HbmfDtcService_GetLin1HvacFanIntErr1  VariantControl=(inherit)
  Ground_1 : Ground
  Ground_2 : Ground
  ErrFlag : ArgOut  OutDataTypeStr=Enum: DTCState  ArgumentName=ErrFlag
  ERR : ArgOut  Port=2  OutDataTypeStr=Std_ReturnType  ArgumentName=ERR
  wires:
  Ground_1/1 -> ErrFlag/1
  Ground_2/1 -> ERR/1

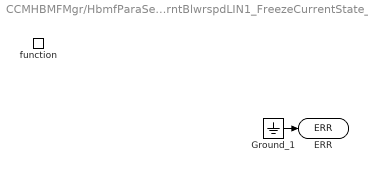
== system CCMHBMFMgr/HbmfParaService/HbmfParaService_FrntBlwrspdLIN1_FreezeCurrentState_sys ==
  function : TriggerPort  TriggerType=function-call  IsSimulinkFunction=on  FunctionName=HbmfParaService_FrntBlwrspdLIN1_FreezeCurrentState  VariantControl=(inherit)
  Ground_1 : Ground
  ERR : ArgOut  OutDataTypeStr=Std_ReturnType  ArgumentName=ERR
  wires:
  Ground_1/1 -> ERR/1

== system CCMHBMFMgr/HbmfParaService/HbmfParaService_FrntBlwrspdLIN1_ReturnControlToECU_sys ==
  function : TriggerPort  TriggerType=function-call  IsSimulinkFunction=on  FunctionName=HbmfParaService_FrntBlwrspdLIN1_ReturnControlToECU  VariantControl=(inherit)
  Ground_1 : Ground
  ERR : ArgOut  OutDataTypeStr=Std_ReturnType  ArgumentName=ERR
  wires:
  Ground_1/1 -> ERR/1

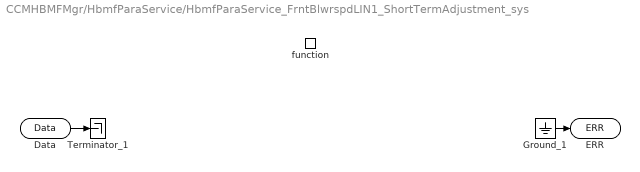
== system CCMHBMFMgr/HbmfParaService/HbmfParaService_FrntBlwrspdLIN1_ShortTermAdjustment_sys ==
  function : TriggerPort  TriggerType=function-call  IsSimulinkFunction=on  FunctionName=HbmfParaService_FrntBlwrspdLIN1_ShortTermAdjustment  VariantControl=(inherit)
  Ground_1 : Ground
  Terminator_1 : Terminator
  Data : ArgIn  OutDataTypeStr=UInt8  ArgumentName=Data
  ERR : ArgOut  OutDataTypeStr=Std_ReturnType  ArgumentName=ERR
  wires:
  Data/1 -> Terminator_1/1
  Ground_1/1 -> ERR/1

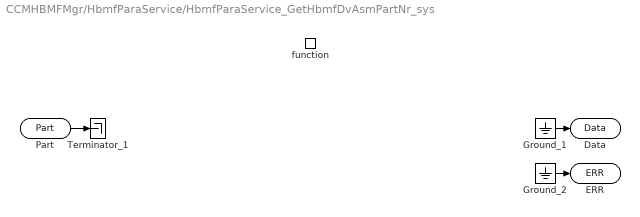
== system CCMHBMFMgr/HbmfParaService/HbmfParaService_GetHbmfDvAsmPartNr_sys ==
  function : TriggerPort  TriggerType=function-call  IsSimulinkFunction=on  FunctionName=HbmfParaService_GetHbmfDvAsmPartNr  VariantControl=(inherit)
  Ground_1 : Ground
  Ground_2 : Ground
  Terminator_1 : Terminator
  Part : ArgIn  OutDataTypeStr=SignalPartType  ArgumentName=Part
  Data : ArgOut  OutDataTypeStr=UInt8  ArgumentName=Data
  ERR : ArgOut  Port=2  OutDataTypeStr=Std_ReturnType  ArgumentName=ERR
  wires:
  Part/1 -> Terminator_1/1
  Ground_1/1 -> Data/1
  Ground_2/1 -> ERR/1

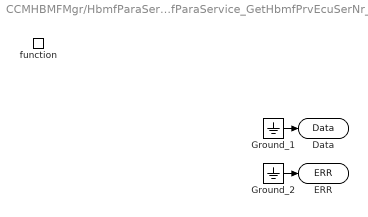
== system CCMHBMFMgr/HbmfParaService/HbmfParaService_GetHbmfPrvEcuSerNr_sys ==
  function : TriggerPort  TriggerType=function-call  IsSimulinkFunction=on  FunctionName=HbmfParaService_GetHbmfPrvEcuSerNr  VariantControl=(inherit)
  Ground_1 : Ground
  Ground_2 : Ground
  Data : ArgOut  OutDataTypeStr=UInt8  ArgumentName=Data
  ERR : ArgOut  Port=2  OutDataTypeStr=Std_ReturnType  ArgumentName=ERR
  wires:
  Ground_1/1 -> Data/1
  Ground_2/1 -> ERR/1
